simulink model "ClimaDefrstFlowMgr"  (R2022b)
settings: Solver=FixedStepDiscrete  StartTime=0.0  StopTime=10.0  FixedStep=1  RelTol=1e-3
settings Model: Creator=blue.ge  LastModifiedBy=blue.ge
settings DataIOCC: SaveFormat=StructureWithTime
settings OptimizationCC: DefaultParameterBehavior=Inlined  EnhancedBackFolding=on  PassReuseOutputArgsAs=Individual arguments  ZeroExternalMemoryAtStartup=off  ZeroInternalMemoryAtStartup=off  OptimizeBlockOrder=speed  DifferentSizesBufferReuse=on  OptimizationCustomize=off  EfficientTunableParamExpr=on
settings DebuggingCC: ParameterTunabilityLossMsg=error
settings ModelReferenceCC: ModelReferenceNumInstancesAllowed=Single
settings SFSimCC: MATLABDynamicMemAlloc=off
settings RTWCC: Description=AUTOSAR  SystemTargetFile=autosar.tlc  GenCodeOnly=on  TemplateMakefile=ert_default_tmf
settings CodeAppCC: ForceParamTrailComments=on  IgnoreCustomStorageClasses=off  OperatorAnnotations=on  SimulinkDataObjDesc=on  SFDataObjDesc=on  InsertBlockDesc=on
settings STFCustomTargetCC: TargetLangStandard=C99 (ISO)  MultiwordLength=256  ParMdlRefBuildCompliant=on  CompOptLevelCompliant=on  IncludeMdlTerminateFcn=off  CombineOutputUpdateFcns=on  SuppressErrorStatus=on  ERTCustomFileBanners=on  SupportNonFinite=off  SupportComplex=off  SupportContinuousTime=off  SupportNonInlinedSFcns=off  RemoveResetFunc=on  MATLABClassNameForMDSCustomization=Simulink.SoftwareTarget.ERTCustomization  AutosarCompliant=on  UseToolchainInfoCompliant=on  LookupTableObjectStructAxisOrder=2,1,3,4,...  LUTObjectStructOrderEvenSpacing=Size,Table,Breakpoints  SystemTargetFile=autosar.tlc
settings ERTTargetCC: TargetLangStandard=C99 (ISO)  ConcurrentExecutionCompliant=off  IncludeMdlTerminateFcn=off  SuppressErrorStatus=on  SupportNonFinite=off  SupportComplex=off  ModelStepFunctionPrototypeControlCompliant=off  CPPClassGenCompliant=off  AutosarCompliant=on  LookupTableObjectStructAxisOrder=2,1,3,4,...  LUTObjectStructOrderEvenSpacing=Size,Table,Breakpoints  InlinedParameterPlacement=NonHierarchical  ERTCustomFileTemplate=example_file_process.tlc

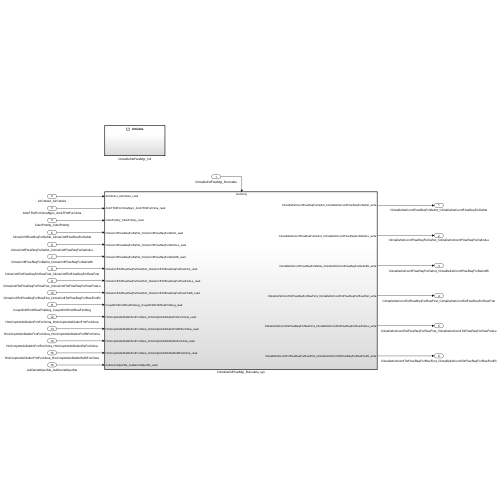
[thumbnail: Simulink's stored preview of the top-level diagram]
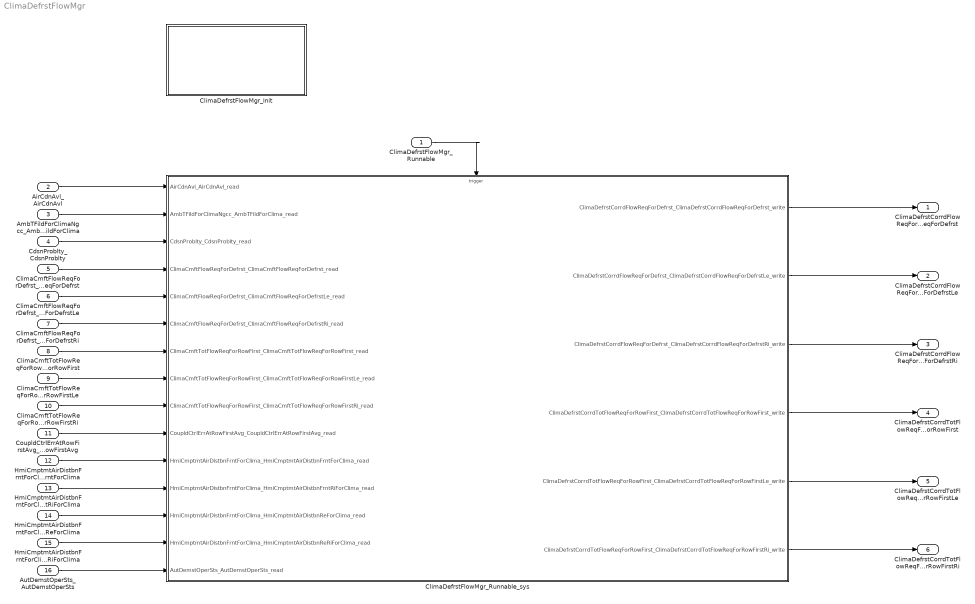
== system ClimaDefrstFlowMgr ==
  ClimaDefrstFlowMgr_Runnable : Inport  SampleTime=1  OutputFunctionCall=on
  AirCdnAvl_AirCdnAvl : Inport  Port=2  OutDataTypeStr=Boolean  PortDimensions=1
  AmbTFildForClimaNgcc_AmbTFildForClima : Inport  Port=3  OutDataTypeStr=T30  PortDimensions=1
  CdsnProblty_CdsnProblty : Inport  Port=4  OutDataTypeStr=Fac30  PortDimensions=1
  ClimaCmftFlowReqForDefrst_ClimaCmftFlowReqForDefrst : Inport  Port=5  OutDataTypeStr=AirFlow30  PortDimensions=1
  ClimaCmftFlowReqForDefrst_ClimaCmftFlowReqForDefrstLe : Inport  Port=6  OutDataTypeStr=AirFlow30  PortDimensions=1
  ClimaCmftFlowReqForDefrst_ClimaCmftFlowReqForDefrstRi : Inport  Port=7  OutDataTypeStr=AirFlow30  PortDimensions=1
  ClimaCmftTotFlowReqForRowFirst_ClimaCmftTotFlowReqForRowFirst : Inport  Port=8  OutDataTypeStr=AirFlow30  PortDimensions=1
  ClimaCmftTotFlowReqForRowFirst_ClimaCmftTotFlowReqForRowFirstLe : Inport  Port=9  OutDataTypeStr=AirFlow30  PortDimensions=1
  ClimaCmftTotFlowReqForRowFirst_ClimaCmftTotFlowReqForRowFirstRi : Inport  Port=10  OutDataTypeStr=AirFlow30  PortDimensions=1
  CoupldCtrlErrAtRowFirstAvg_CoupldCtrlErrAtRowFirstAvg : Inport  Port=11  OutDataTypeStr=T30  PortDimensions=1
  HmiCmptmtAirDistbnFrntForClima_HmiCmptmtAirDistbnFrntForClima : Inport  Port=12  OutDataTypeStr=Enum: HmiCmptmtAirDistbnFrnt  PortDimensions=1
  HmiCmptmtAirDistbnFrntForClima_HmiCmptmtAirDistbnFrntRiForClima : Inport  Port=13  OutDataTypeStr=Enum: HmiCmptmtAirDistbnFrnt  PortDimensions=1
  HmiCmptmtAirDistbnFrntForClima_HmiCmptmtAirDistbnReForClima : Inport  Port=14  OutDataTypeStr=Enum: HmiCmptmtAirDistbnFrnt  PortDimensions=1
  HmiCmptmtAirDistbnFrntForClima_HmiCmptmtAirDistbnReRiForClima : Inport  Port=15  OutDataTypeStr=Enum: HmiCmptmtAirDistbnFrnt  PortDimensions=1
  AutDemstOperSts_AutDemstOperSts : Inport  Port=16  OutDataTypeStr=Enum: OnOff1  PortDimensions=1
  ClimaDefrstFlowMgr_Init : Subsystem
  ClimaDefrstFlowMgr_Runnable_sys : Subsystem
  ClimaDefrstCorrdFlowReqForDefrst_ClimaDefrstCorrdFlowReqForDefrst : Outport  OutDataTypeStr=AirFlow30  PortDimensions=1
  ClimaDefrstCorrdFlowReqForDefrst_ClimaDefrstCorrdFlowReqForDefrstLe : Outport  Port=2  OutDataTypeStr=AirFlow30  PortDimensions=1
  ClimaDefrstCorrdFlowReqForDefrst_ClimaDefrstCorrdFlowReqForDefrstRi : Outport  Port=3  OutDataTypeStr=AirFlow30  PortDimensions=1
  ClimaDefrstCorrdTotFlowReqForRowFirst_ClimaDefrstCorrdTotFlowReqForRowFirst : Outport  Port=4  OutDataTypeStr=AirFlow30  PortDimensions=1
  ClimaDefrstCorrdTotFlowReqForRowFirst_ClimaDefrstCorrdTotFlowReqForRowFirstLe : Outport  Port=5  OutDataTypeStr=AirFlow30  PortDimensions=1
  ClimaDefrstCorrdTotFlowReqForRowFirst_ClimaDefrstCorrdTotFlowReqForRowFirstRi : Outport  Port=6  OutDataTypeStr=AirFlow30  PortDimensions=1
  wires:
  AirCdnAvl_AirCdnAvl/1 -> ClimaDefrstFlowMgr_Runnable_sys/1(AirCdnAvl_AirCdnAvl_read)
  AmbTFildForClimaNgcc_AmbTFildForClima/1 -> ClimaDefrstFlowMgr_Runnable_sys/2(AmbTFildForClimaNgcc_AmbTFildForClima_read)
  CdsnProblty_CdsnProblty/1 -> ClimaDefrstFlowMgr_Runnable_sys/3(CdsnProblty_CdsnProblty_read)
  ClimaCmftFlowReqForDefrst_ClimaCmftFlowReqForDefrst/1 -> ClimaDefrstFlowMgr_Runnable_sys/4(ClimaCmftFlowReqForDefrst_ClimaCmftFlowReqForDefrst_read)
  ClimaCmftFlowReqForDefrst_ClimaCmftFlowReqForDefrstLe/1 -> ClimaDefrstFlowMgr_Runnable_sys/5(ClimaCmftFlowReqForDefrst_ClimaCmftFlowReqForDefrstLe_read)
  ClimaCmftFlowReqForDefrst_ClimaCmftFlowReqForDefrstRi/1 -> ClimaDefrstFlowMgr_Runnable_sys/6(ClimaCmftFlowReqForDefrst_ClimaCmftFlowReqForDefrstRi_read)
  ClimaCmftTotFlowReqForRowFirst_ClimaCmftTotFlowReqForRowFirst/1 -> ClimaDefrstFlowMgr_Runnable_sys/7(ClimaCmftTotFlowReqForRowFirst_ClimaCmftTotFlowReqForRowFirst_read)
  ClimaCmftTotFlowReqForRowFirst_ClimaCmftTotFlowReqForRowFirstLe/1 -> ClimaDefrstFlowMgr_Runnable_sys/8(ClimaCmftTotFlowReqForRowFirst_ClimaCmftTotFlowReqForRowFirstLe_read)
  ClimaCmftTotFlowReqForRowFirst_ClimaCmftTotFlowReqForRowFirstRi/1 -> ClimaDefrstFlowMgr_Runnable_sys/9(ClimaCmftTotFlowReqForRowFirst_ClimaCmftTotFlowReqForRowFirstRi_read)
  CoupldCtrlErrAtRowFirstAvg_CoupldCtrlErrAtRowFirstAvg/1 -> ClimaDefrstFlowMgr_Runnable_sys/10(CoupldCtrlErrAtRowFirstAvg_CoupldCtrlErrAtRowFirstAvg_read)
  HmiCmptmtAirDistbnFrntForClima_HmiCmptmtAirDistbnFrntForClima/1 -> ClimaDefrstFlowMgr_Runnable_sys/11(HmiCmptmtAirDistbnFrntForClima_HmiCmptmtAirDistbnFrntForClima_read)
  HmiCmptmtAirDistbnFrntForClima_HmiCmptmtAirDistbnFrntRiForClima/1 -> ClimaDefrstFlowMgr_Runnable_sys/12(HmiCmptmtAirDistbnFrntForClima_HmiCmptmtAirDistbnFrntRiForClima_read)
  HmiCmptmtAirDistbnFrntForClima_HmiCmptmtAirDistbnReForClima/1 -> ClimaDefrstFlowMgr_Runnable_sys/13(HmiCmptmtAirDistbnFrntForClima_HmiCmptmtAirDistbnReForClima_read)
  HmiCmptmtAirDistbnFrntForClima_HmiCmptmtAirDistbnReRiForClima/1 -> ClimaDefrstFlowMgr_Runnable_sys/14(HmiCmptmtAirDistbnFrntForClima_HmiCmptmtAirDistbnReRiForClima_read)
  AutDemstOperSts_AutDemstOperSts/1 -> ClimaDefrstFlowMgr_Runnable_sys/15(AutDemstOperSts_AutDemstOperSts_read)
  ClimaDefrstFlowMgr_Runnable_sys/1(ClimaDefrstCorrdFlowReqForDefrst_ClimaDefrstCorrdFlowReqForDefrst_write) -> ClimaDefrstCorrdFlowReqForDefrst_ClimaDefrstCorrdFlowReqForDefrst/1
  ClimaDefrstFlowMgr_Runnable_sys/2(ClimaDefrstCorrdFlowReqForDefrst_ClimaDefrstCorrdFlowReqForDefrstLe_write) -> ClimaDefrstCorrdFlowReqForDefrst_ClimaDefrstCorrdFlowReqForDefrstLe/1
  ClimaDefrstFlowMgr_Runnable_sys/3(ClimaDefrstCorrdFlowReqForDefrst_ClimaDefrstCorrdFlowReqForDefrstRi_write) -> ClimaDefrstCorrdFlowReqForDefrst_ClimaDefrstCorrdFlowReqForDefrstRi/1
  ClimaDefrstFlowMgr_Runnable_sys/4(ClimaDefrstCorrdTotFlowReqForRowFirst_ClimaDefrstCorrdTotFlowReqForRowFirst_write) -> ClimaDefrstCorrdTotFlowReqForRowFirst_ClimaDefrstCorrdTotFlowReqForRowFirst/1
  ClimaDefrstFlowMgr_Runnable_sys/5(ClimaDefrstCorrdTotFlowReqForRowFirst_ClimaDefrstCorrdTotFlowReqForRowFirstLe_write) -> ClimaDefrstCorrdTotFlowReqForRowFirst_ClimaDefrstCorrdTotFlowReqForRowFirstLe/1
  ClimaDefrstFlowMgr_Runnable_sys/6(ClimaDefrstCorrdTotFlowReqForRowFirst_ClimaDefrstCorrdTotFlowReqForRowFirstRi_write) -> ClimaDefrstCorrdTotFlowReqForRowFirst_ClimaDefrstCorrdTotFlowReqForRowFirstRi/1
  ClimaDefrstFlowMgr_Runnable/1 -> ClimaDefrstFlowMgr_Runnable_sys/Trigger

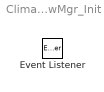
== system ClimaDefrstFlowMgr/ClimaDefrstFlowMgr_Init ==
  Event Listener : EventListener  EventName=reset

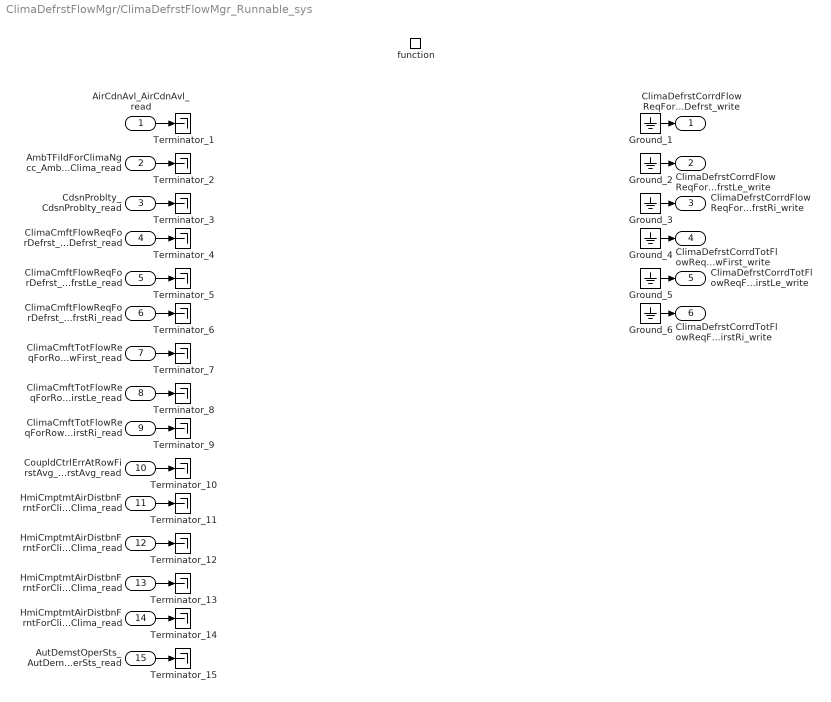
== system ClimaDefrstFlowMgr/ClimaDefrstFlowMgr_Runnable_sys ==
  AirCdnAvl_AirCdnAvl_read : Inport
  AmbTFildForClimaNgcc_AmbTFildForClima_read : Inport  Port=2
  CdsnProblty_CdsnProblty_read : Inport  Port=3
  ClimaCmftFlowReqForDefrst_ClimaCmftFlowReqForDefrst_read : Inport  Port=4
  ClimaCmftFlowReqForDefrst_ClimaCmftFlowReqForDefrstLe_read : Inport  Port=5
  ClimaCmftFlowReqForDefrst_ClimaCmftFlowReqForDefrstRi_read : Inport  Port=6
  ClimaCmftTotFlowReqForRowFirst_ClimaCmftTotFlowReqForRowFirst_read : Inport  Port=7
  ClimaCmftTotFlowReqForRowFirst_ClimaCmftTotFlowReqForRowFirstLe_read : Inport  Port=8
  ClimaCmftTotFlowReqForRowFirst_ClimaCmftTotFlowReqForRowFirstRi_read : Inport  Port=9
  CoupldCtrlErrAtRowFirstAvg_CoupldCtrlErrAtRowFirstAvg_read : Inport  Port=10
  HmiCmptmtAirDistbnFrntForClima_HmiCmptmtAirDistbnFrntForClima_read : Inport  Port=11
  HmiCmptmtAirDistbnFrntForClima_HmiCmptmtAirDistbnFrntRiForClima_read : Inport  Port=12
  HmiCmptmtAirDistbnFrntForClima_HmiCmptmtAirDistbnReForClima_read : Inport  Port=13
  HmiCmptmtAirDistbnFrntForClima_HmiCmptmtAirDistbnReRiForClima_read : Inport  Port=14
  AutDemstOperSts_AutDemstOperSts_read : Inport  Port=15
  function : TriggerPort  TriggerType=function-call  VariantControl=(inherit)  SampleTimeType=periodic  SampleTime=-1
  Ground_{1..6} (6 alike) : Ground
  Terminator_{1..15} (15 alike) : Terminator
  ClimaDefrstCorrdFlowReqForDefrst_ClimaDefrstCorrdFlowReqForDefrst_write : Outport
      settings: EnsureOutportIsVirtual=on
  ClimaDefrstCorrdFlowReqForDefrst_ClimaDefrstCorrdFlowReqForDefrstLe_write : Outport  Port=2
      settings: EnsureOutportIsVirtual=on
  ClimaDefrstCorrdFlowReqForDefrst_ClimaDefrstCorrdFlowReqForDefrstRi_write : Outport  Port=3
      settings: EnsureOutportIsVirtual=on
  ClimaDefrstCorrdTotFlowReqForRowFirst_ClimaDefrstCorrdTotFlowReqForRowFirst_write : Outport  Port=4
      settings: EnsureOutportIsVirtual=on
  ClimaDefrstCorrdTotFlowReqForRowFirst_ClimaDefrstCorrdTotFlowReqForRowFirstLe_write : Outport  Port=5
      settings: EnsureOutportIsVirtual=on
  ClimaDefrstCorrdTotFlowReqForRowFirst_ClimaDefrstCorrdTotFlowReqForRowFirstRi_write : Outport  Port=6
      settings: EnsureOutportIsVirtual=on
  wires:
  AirCdnAvl_AirCdnAvl_read/1 -> Terminator_1/1
  AmbTFildForClimaNgcc_AmbTFildForClima_read/1 -> Terminator_2/1
  CdsnProblty_CdsnProblty_read/1 -> Terminator_3/1
  ClimaCmftFlowReqForDefrst_ClimaCmftFlowReqForDefrst_read/1 -> Terminator_4/1
  ClimaCmftFlowReqForDefrst_ClimaCmftFlowReqForDefrstLe_read/1 -> Terminator_5/1
  ClimaCmftFlowReqForDefrst_ClimaCmftFlowReqForDefrstRi_read/1 -> Terminator_6/1
  ClimaCmftTotFlowReqForRowFirst_ClimaCmftTotFlowReqForRowFirst_read/1 -> Terminator_7/1
  ClimaCmftTotFlowReqForRowFirst_ClimaCmftTotFlowReqForRowFirstLe_read/1 -> Terminator_8/1
  ClimaCmftTotFlowReqForRowFirst_ClimaCmftTotFlowReqForRowFirstRi_read/1 -> Terminator_9/1
  CoupldCtrlErrAtRowFirstAvg_CoupldCtrlErrAtRowFirstAvg_read/1 -> Terminator_10/1
  HmiCmptmtAirDistbnFrntForClima_HmiCmptmtAirDistbnFrntForClima_read/1 -> Terminator_11/1
  HmiCmptmtAirDistbnFrntForClima_HmiCmptmtAirDistbnFrntRiForClima_read/1 -> Terminator_12/1
  HmiCmptmtAirDistbnFrntForClima_HmiCmptmtAirDistbnReForClima_read/1 -> Terminator_13/1
  HmiCmptmtAirDistbnFrntForClima_HmiCmptmtAirDistbnReRiForClima_read/1 -> Terminator_14/1
  AutDemstOperSts_AutDemstOperSts_read/1 -> Terminator_15/1
  Ground_1/1 -> ClimaDefrstCorrdFlowReqForDefrst_ClimaDefrstCorrdFlowReqForDefrst_write/1
  Ground_2/1 -> ClimaDefrstCorrdFlowReqForDefrst_ClimaDefrstCorrdFlowReqForDefrstLe_write/1
  Ground_3/1 -> ClimaDefrstCorrdFlowReqForDefrst_ClimaDefrstCorrdFlowReqForDefrstRi_write/1
  Ground_4/1 -> ClimaDefrstCorrdTotFlowReqForRowFirst_ClimaDefrstCorrdTotFlowReqForRowFirst_write/1
  Ground_5/1 -> ClimaDefrstCorrdTotFlowReqForRowFirst_ClimaDefrstCorrdTotFlowReqForRowFirstLe_write/1
  Ground_6/1 -> ClimaDefrstCorrdTotFlowReqForRowFirst_ClimaDefrstCorrdTotFlowReqForRowFirstRi_write/1
